simulink model "TosAeb"  (R2021a)
settings: Solver=FixedStepDiscrete  StartTime=0.0  StopTime=inf  FixedStep=0.01  RelTol=1e-3
undefined symbols (not defined in the file or in its folder): ACCModSts, AccSts1, AEB_ObjectMovingOrientation, AEB_ObjectMovingState, CN_BTCActive, CN_CurrentGearR, CN_CurrentGearR_DHT, CN_PTCActive, CN_VDCActive, CN_VSC_EspOff, CN_VSC_FCW_AEB_Error, CN_VSC_FCW_AEB_NoDispay, CN_VSC_FCW_AEB_PerfmDgdtn, CN_VSC_FCW_AEB_TempUnAvlbl, CN_VSC_VehStandstill, CNaccelAvoidMax, CNaccelAvoidMin, CNbrakePedalPress, CNcomfortStrLeftAccelMax, CNcomfortStrLeftAccelMin, CNcomfortStrRightAccelMax, CNcomfortStrRightAccelMin, CNDrvDoorOpen, CNDrvModeEco, CNDrvModeNorm, CNDrvModeOther, CNDrvModeSport, CNDrvSBRreminder, CNNull, CNobjInvalidID, CNPassDoorOpen, CNPassSBRreminder, CNPEBSAccelNegInf, CNPEBSAccelNull, CNPEBSTimeInf, CNPEBSTimeNull, CNSTOPMAX, CNSTOPNULL, CNsubjWidth, CNSwtOFF, CNSwtON, CNTTCMAX, CNTTCNULL, CNVehConfigAEBCycNone, CNVehConfigAEBNone, CNVehConfigAEBPedNone, CNVehConfigFCWNone, CNVehConfigJANone, CollisionSeverity, FALSE, FCWSnvty, JACycObjCalcInfoDefaultParams, JAPedObjCalcInfoDefaultParams, JAVehObjCalcInfoDefaultParams, latCycObjCalcInfoDefaultParams, latPedObjCalcInfoDefaultParams, longObjCalcInfoDefaultParams, ModSts, movingStatus, ObjectType ... (+1112)
libraries: simulink x3031, AEBSFunctionLib x50, Ta_Library x33, AEBSStateMachineLib x23, Mylibrary x12, simulink_extras x7, ObjSelect_lib x3, dspstat3 x2
(part 22 of 102)

== system TosAeb/AEB_Objsel/TA_Processor/PreProcessor/VehiclePreProcessor/PathEstimn_Calculate/For Iterator PahEstimn/SetVehicleStateAtT0 ==
  VehSelf : Inport
  Curvature : Inport  Port=2
  CurvatureRate : Inport  Port=3
  Index : Inport  Port=4
  VehicleMotionT1 : Inport  Port=5
  Bus Creator1 : BusCreator  Inputs=11
  Bus Selector : BusSelector  OutputSignals=Veh_LgtSpd_mps,Veh_LgtAccel_mps2
  Bus Selector1 : BusSelector  OutputSignals=PositionLongitudinal
  Bus Selector10 : BusSelector  OutputSignals=HeadingAngle
  Bus Selector2 : BusSelector  OutputSignals=VelocityLateral
  Bus Selector3 : BusSelector  OutputSignals=A
  Bus Selector4 : BusSelector  OutputSignals=AccelerationLongitudinal
  Bus Selector5 : BusSelector  OutputSignals=PositionLateral
  Bus Selector6 : BusSelector  OutputSignals=VelocityLateral
  Bus Selector7 : BusSelector  OutputSignals=AccelerationLateral
  Bus Selector8 : BusSelector  OutputSignals=Speed
  Bus Selector9 : BusSelector  OutputSignals=Curvature
  Compare To Constant : simulink/Logic and Bit Operations/Compare To Constant  relop===  const=1  OutDataTypeStr=boolean
  Constant{,1..4} (5 alike) : Constant  Value=single(0)
  Multiport Switch : MultiPortSwitch  Inputs=4
  Product : Product  Inputs=3
  Switch{,1..9} (10 alike) : Switch  Criteria=u2 ~= 0
  Unary Minus : UnaryMinus
  Unit Delay{,1..9} (10 alike) : UnitDelay  SampleTime=-1  HasFrameUpgradeWarning=on
  VehicleMotionT0 : Outport
  wires:
  Index/1 -> [Index], Compare To Constant/1
  Compare To Constant/1 -> [InitialLoop]
  Constant/1 -> Switch/1
  [InitialLoop] -> Switch/2
  VehicleMotionT1/1 -> [VehicleMotionT1]
  Bus Selector1/1 -> Unit Delay/1  "<PositionLongitudinal>"
  Unit Delay/1 -> Switch/3
  [InitialLoop] -> Switch1/2
  [VLgt] -> Switch1/1
  Unit Delay1/1 -> Switch1/3
  [InitialLoop] -> Switch2/2
  [ALgt] -> Switch2/1
  Unit Delay2/1 -> Switch2/3
  [VehicleMotionT1] -> Bus Selector2/1  "VelocityLongItudal"
  [VehicleMotionT1] -> Bus Selector3/1  "A"
  [InitialLoop] -> Switch3/2
  [ALgt] -> Switch3/1
  [VehicleMotionT1] -> Bus Selector4/1  "ALgt"
  Bus Selector4/1 -> Unit Delay3/1  "<AccelerationLongitudinal>"
  Unit Delay3/1 -> Switch3/3
  [VehicleMotionT1] -> Bus Selector5/1  "PosnLat"
  Bus Selector5/1 -> Unit Delay4/1  "<PositionLateral>"
  Unit Delay4/1 -> Switch4/3
  [InitialLoop] -> Switch4/2
  Constant1/1 -> Switch4/1
  [VehicleMotionT1] -> Bus Selector6/1  "VLat"
  Unit Delay5/1 -> Switch5/3
  [InitialLoop] -> Switch5/2
  Constant2/1 -> Switch5/1
  Product/1 -> Switch6/1
  [InitialLoop] -> Switch6/2
  Unit Delay6/1 -> Switch6/3
  [VehicleMotionT1] -> Bus Selector7/1  "ALat"
  [VLgt] -> Product/2, Product/3
  Curvature/1 -> Switch9/1, Product/1
  [Index] -> Multiport Switch/1
  CurvatureRate/1 -> Multiport Switch/2, Unary Minus/1
  Unary Minus/1 -> Multiport Switch/3
  Constant3/1 -> Multiport Switch/4, Multiport Switch/5
  Constant4/1 -> Switch7/1
  [InitialLoop] -> Switch7/2
  Bus Selector7/1 -> Unit Delay6/1  "<AccelerationLateral>"
  [VehicleMotionT1] -> Bus Selector10/1  "HeadingAngle"
  Bus Selector10/1 -> Unit Delay9/1  "<HeadingAngle>"
  [VLgt] -> Switch8/1
  [InitialLoop] -> Switch8/2
  [VehicleMotionT1] -> Bus Selector8/1  "Speed"
  Bus Selector8/1 -> Unit Delay7/1  "<Speed>"
  [InitialLoop] -> Switch9/2
  [VehicleMotionT1] -> Bus Selector9/1  "Curvature"
  Bus Selector9/1 -> Unit Delay8/1  "<Curvature>"
  Switch7/1 -> Bus Creator1/11  "HeadingAngle"
  Switch2/1 -> Bus Creator1/3  "A"
  Switch3/1 -> Bus Creator1/4  "AccelerationLongitudinal"
  Switch4/1 -> Bus Creator1/5  "PositionLateral"
  Switch6/1 -> Bus Creator1/7  "AccelerationLateral"
  Switch8/1 -> Bus Creator1/8  "Speed"
  Switch9/1 -> Bus Creator1/9  "Curvature"
  Multiport Switch/1 -> Bus Creator1/10  "CurvatureRate"
  Switch/1 -> Bus Creator1/1  "PositionLongitudinal"
  Switch1/1 -> Bus Creator1/2  "VelocityLongitudinal"
  Switch5/1 -> Bus Creator1/6  "VelocityLateral"
  Bus Creator1/1 -> VehicleMotionT0/1  "VehicleMotionT0"
  VehSelf/1 -> Bus Selector/1  "1"
  [VehicleMotionT1] -> Bus Selector1/1  "PositionLongItudal"
  Bus Selector2/1 -> Unit Delay1/1  "<VelocityLateral>"
  Bus Selector3/1 -> Unit Delay2/1  "<A>"
  Bus Selector6/1 -> Unit Delay5/1  "<VelocityLateral>"
  Bus Selector/1 -> [VLgt]  "<Veh_LgtSpd_mps>"
  Bus Selector/2 -> [ALgt]  "<Veh_LgtAccel_mps2>"
  Unit Delay7/1 -> Switch8/3
  Unit Delay8/1 -> Switch9/3
  Unit Delay9/1 -> Switch7/3

== system TosAeb/ELK_Objsel/Target_Selection/If Action Subsystem/ELK_TargetSelection/If Action Threat_Assessment_Left/First_Order_Lag_Filter/Subsystem ==
  note: Filter Gain = T / (T + t)
      | T=> Loop time and
      | t => time constant
  CL_TIME : Inport
  time_constant : Inport  Port=2
  Product : Product  Description=_GWMAB30P4.LKAFN.000_144_  Inputs=*/  InputSameDT=on  OutDataTypeStr=Inherit: Same as first input
  Sum2 : Sum  Description=_GWMAB30P4.LKAFN.000_145_  InputSameDT=on  OutDataTypeStr=Inherit: Same as first input
  Gain : Outport
  wires:
  time_constant/1 -> Sum2/2
  CL_TIME/1 -> Sum2/1, Product/1
  Product/1 -> Gain/1
  Sum2/1 -> Product/2

== system TosAeb/ELK_Objsel/Target_Selection/If Action Subsystem/ELK_TargetSelection/If Action Threat_Assessment_Left/First_Order_Lag_Filter1/Subsystem ==
  note: Filter Gain = T / (T + t)
      | T=> Loop time and
      | t => time constant
  CL_TIME : Inport
  time_constant : Inport  Port=2
  Product : Product  Description=_GWMAB30P4.LKAFN.000_144_  Inputs=*/  InputSameDT=on  OutDataTypeStr=Inherit: Same as first input
  Sum2 : Sum  Description=_GWMAB30P4.LKAFN.000_145_  InputSameDT=on  OutDataTypeStr=Inherit: Same as first input
  Gain : Outport
  wires:
  Sum2/1 -> Product/2
  Product/1 -> Gain/1
  CL_TIME/1 -> Product/1, Sum2/1
  time_constant/1 -> Sum2/2

== system TosAeb/ELK_Objsel/Target_Selection/If Action Subsystem/ELK_TargetSelection/If Action Threat_Assessment_Left1/First_Order_Lag_Filter/Subsystem ==
  note: Filter Gain = T / (T + t)
      | T=> Loop time and
      | t => time constant
  CL_TIME : Inport
  time_constant : Inport  Port=2
  Product : Product  Description=_GWMAB30P4.LKAFN.000_144_  Inputs=*/  InputSameDT=on  OutDataTypeStr=Inherit: Same as first input
  Sum2 : Sum  Description=_GWMAB30P4.LKAFN.000_145_  InputSameDT=on  OutDataTypeStr=Inherit: Same as first input
  Gain : Outport
  wires:
  Sum2/1 -> Product/2
  Product/1 -> Gain/1
  CL_TIME/1 -> Product/1, Sum2/1
  time_constant/1 -> Sum2/2

== system TosAeb/ELK_Objsel/Target_Selection/If Action Subsystem/ELK_TargetSelection/If Action Threat_Assessment_Left1/First_Order_Lag_Filter1/Subsystem ==
  note: Filter Gain = T / (T + t)
      | T=> Loop time and
      | t => time constant
  CL_TIME : Inport
  time_constant : Inport  Port=2
  Product : Product  Description=_GWMAB30P4.LKAFN.000_144_  Inputs=*/  InputSameDT=on  OutDataTypeStr=Inherit: Same as first input
  Sum2 : Sum  Description=_GWMAB30P4.LKAFN.000_145_  InputSameDT=on  OutDataTypeStr=Inherit: Same as first input
  Gain : Outport
  wires:
  time_constant/1 -> Sum2/2
  CL_TIME/1 -> Sum2/1, Product/1
  Product/1 -> Gain/1
  Sum2/1 -> Product/2

== system TosAeb/ELK_Objsel/Target_Selection/If Action Subsystem/ELK_TargetSelection/If Action Threat_Assessment_Right/First_Order_Lag_Filter/Subsystem ==
  note: Filter Gain = T / (T + t)
      | T=> Loop time and
      | t => time constant
  CL_TIME : Inport
  time_constant : Inport  Port=2
  Product : Product  Description=_GWMAB30P4.LKAFN.000_144_  Inputs=*/  InputSameDT=on  OutDataTypeStr=Inherit: Same as first input
  Sum2 : Sum  Description=_GWMAB30P4.LKAFN.000_145_  InputSameDT=on  OutDataTypeStr=Inherit: Same as first input
  Gain : Outport
  wires:
  time_constant/1 -> Sum2/2
  CL_TIME/1 -> Sum2/1, Product/1
  Product/1 -> Gain/1
  Sum2/1 -> Product/2

== system TosAeb/ELK_Objsel/Target_Selection/If Action Subsystem/ELK_TargetSelection/If Action Threat_Assessment_Right/First_Order_Lag_Filter1/Subsystem ==
  note: Filter Gain = T / (T + t)
      | T=> Loop time and
      | t => time constant
  CL_TIME : Inport
  time_constant : Inport  Port=2
  Product : Product  Description=_GWMAB30P4.LKAFN.000_144_  Inputs=*/  InputSameDT=on  OutDataTypeStr=Inherit: Same as first input
  Sum2 : Sum  Description=_GWMAB30P4.LKAFN.000_145_  InputSameDT=on  OutDataTypeStr=Inherit: Same as first input
  Gain : Outport
  wires:
  Sum2/1 -> Product/2
  Product/1 -> Gain/1
  CL_TIME/1 -> Product/1, Sum2/1
  time_constant/1 -> Sum2/2

== system TosAeb/ELK_Objsel/Target_Selection/If Action Subsystem/ELK_TargetSelection/If Action Threat_Assessment_Right1/First_Order_Lag_Filter/Subsystem ==
  note: Filter Gain = T / (T + t)
      | T=> Loop time and
      | t => time constant
  CL_TIME : Inport
  time_constant : Inport  Port=2
  Product : Product  Description=_GWMAB30P4.LKAFN.000_144_  Inputs=*/  InputSameDT=on  OutDataTypeStr=Inherit: Same as first input
  Sum2 : Sum  Description=_GWMAB30P4.LKAFN.000_145_  InputSameDT=on  OutDataTypeStr=Inherit: Same as first input
  Gain : Outport
  wires:
  Sum2/1 -> Product/2
  Product/1 -> Gain/1
  CL_TIME/1 -> Product/1, Sum2/1
  time_constant/1 -> Sum2/2

== system TosAeb/ELK_Objsel/Target_Selection/If Action Subsystem/ELK_TargetSelection/If Action Threat_Assessment_Right1/First_Order_Lag_Filter1/Subsystem ==
  note: Filter Gain = T / (T + t)
      | T=> Loop time and
      | t => time constant
  CL_TIME : Inport
  time_constant : Inport  Port=2
  Product : Product  Description=_GWMAB30P4.LKAFN.000_144_  Inputs=*/  InputSameDT=on  OutDataTypeStr=Inherit: Same as first input
  Sum2 : Sum  Description=_GWMAB30P4.LKAFN.000_145_  InputSameDT=on  OutDataTypeStr=Inherit: Same as first input
  Gain : Outport
  wires:
  time_constant/1 -> Sum2/2
  CL_TIME/1 -> Sum2/1, Product/1
  Product/1 -> Gain/1
  Sum2/1 -> Product/2

== system TosAeb/FcwAebJa_Step_sys/ALG_AEBSystem/ALG_AEBController/AEB_FuncCondCalcApp/CycFunctionCondCalc/cycAEBfullSuppAborCond/cycAEBfullAborCondNoStandstillCalc ==
  inputSignals : Inport
  Bus Selector : BusSelector  OutputSignals=latCycTgtCalcInfo.latCycObjCalcInfo.latCycObjRawInfo.ObjectID,latCycTgtCalcInfo.latCycObjCalcInfo.latCycObjRawInfo.ObjectTypeCameraConfirmed,latCycTgtCalcInfo.latCycObjCalcInfo.CollisionRiskProb
  Bus Selector1 : BusSelector  OutputSignals=vehCanInfo.SteerWheelAngle,vehCanInfo.SteerWheelSpd,vehStateCalcInfo.VSCPedFuncSuppAborCond.VSCPedAEBfullAborCond,latFuncActiveStateTime.pedAEBfullWorkTime,vehStateCalcInfo.VSCVehStandStill
  Bus Selector2 : BusSelector  OutputSignals=latCycTgtCalcInfo.latCycCollisionAssessInfo.latCollisionSeverity,latCycTgtCalcInfo.latCycObjCalcInfo.CollisionRiskProb,subjInfo.subjVel,latCycTgtCalcInfo.latCycObjCalcInfo.latCycObjRawInfo.dy,latCycTgtCalcInfo.latCycObjCalcInfo.latCycObjRawInfo.dx,latCycTgtCalcInfo.latCycObjCalcInfo.latCycObjRawInfo.ObjectMovingState
  Ground : Ground
  cycAEBfullAborCondFinalJudge : Subsystem
  cycAEBfullObjRelActiveCondCalc : Subsystem
  cycAEBfullSituationRelAborCondCalc : Subsystem
  cycAEBfullVehRelAborCondCalc : Subsystem
  cycAEBfullAborCondNoStandstillFulfilled : Outport
  wires:
  Bus Selector2/2 -> cycAEBfullSituationRelAborCondCalc/3(objLatCollsionRiskProb)  "<CollisionRiskProb>"
  Bus Selector2/3 -> cycAEBfullSituationRelAborCondCalc/4(subjVel)  "<subjVel>"
  Bus Selector2/4 -> cycAEBfullSituationRelAborCondCalc/5(dy)  "<dy>"
  Bus Selector2/5 -> cycAEBfullSituationRelAborCondCalc/6(dx)  "<dx>"
  Bus Selector2/6 -> cycAEBfullSituationRelAborCondCalc/7(ObjectMovingState)  "<ObjectMovingState>"
  cycAEBfullObjRelActiveCondCalc/1(cycAEBfullObjRelAborCondFulfilled) -> cycAEBfullAborCondFinalJudge/1(cycAEBfullObjRelAborCondFulfilled)  "AEB_cycAEBfullObjRelAborCond"
  cycAEBfullVehRelAborCondCalc/1(cycAEBfullVehRelAborCondFulfilled) -> cycAEBfullAborCondFinalJudge/2(cycAEBfullVehRelAborCondFulfilled)  "AEB_cycAEBfullVehRelAborCond"
  cycAEBfullSituationRelAborCondCalc/1(cycAEBfullSituationRelAborCondFulfilled) -> cycAEBfullAborCondFinalJudge/3(cycAEBfullSituationRelAborCondFulfilled)  "AEB_cycAEBfullSitRelAborCond"
  cycAEBfullAborCondFinalJudge/1(cycAEBfullAborCondNoStandstillFulfilled) -> cycAEBfullAborCondNoStandstillFulfilled/1
  inputSignals/1 -> Bus Selector/1, Bus Selector2/1, Bus Selector1/1
  Bus Selector1/1 -> cycAEBfullVehRelAborCondCalc/1(SteerWheelAngle)  "<SteerWheelAngle>"
  Bus Selector1/2 -> cycAEBfullVehRelAborCondCalc/2(SteerWheelSpd)  "<SteerWheelSpd>"
  Bus Selector1/3 -> cycAEBfullVehRelAborCondCalc/3(vehStateCalcAEBfullAborFlg)  "<VSCPedAEBfullAborCond>"
  Bus Selector1/4 -> cycAEBfullVehRelAborCondCalc/4(cycAEBfullWorkTime)  "<pedAEBfullWorkTime>"
  Bus Selector1/5 -> cycAEBfullVehRelAborCondCalc/5(vehStateCalcStandstill)  "<VSCVehStandStill>"
  Ground/1 -> cycAEBfullSituationRelAborCondCalc/1(cycVehVelReduced)
  Bus Selector2/1 -> cycAEBfullSituationRelAborCondCalc/2(cycCollisionSeverity)  "<latCollisionSeverity>"
  Bus Selector/1 -> cycAEBfullObjRelActiveCondCalc/1(ObjectID)  "<ObjectID>"
  Bus Selector/2 -> cycAEBfullObjRelActiveCondCalc/2(objIsFusionTarget)  "<ObjectTypeCameraConfirmed>"
  Bus Selector/3 -> cycAEBfullObjRelActiveCondCalc/3(latCollsionRiskProb)  "<CollisionRiskProb>"

== system TosAeb/FcwAebJa_Step_sys/ALG_AEBSystem/ALG_AEBController/AEB_FuncCondCalcApp/CycFunctionCondCalc/cycAEBfullSuppAborCond/cycAEBfullAborCondStandstillCalc ==
  inputSignals : Inport
  Bus Selector : BusSelector  OutputSignals=subjInfo.subjVel,vehCanInfo.SteerWheelAngle,vehCanInfo.SteerWheelSpd,vehStateCalcInfo.VSCCycFuncSuppAborCond.VSCcycAEBfullAborCond,latFuncActiveStateTime.cycAEBfullWorkTime,vehStateCalcInfo.VSCVehStandStill
  cycAEBfullVehRelAborCondCalc : Subsystem
  cycAEBfullAborCondStandstillFulfilled : Outport
  wires:
  cycAEBfullVehRelAborCondCalc/1(cycAEBfullVehRelAborCondFulfilled) -> cycAEBfullAborCondStandstillFulfilled/1
  inputSignals/1 -> Bus Selector/1
  Bus Selector/3 -> cycAEBfullVehRelAborCondCalc/3(SteerWheelSpd)  "<SteerWheelSpd>"
  Bus Selector/2 -> cycAEBfullVehRelAborCondCalc/2(SteerWheelAngle)  "<SteerWheelAngle>"
  Bus Selector/4 -> cycAEBfullVehRelAborCondCalc/4(vehStateCalcAEBfullAborFlg)  "<VSCcycAEBfullAborCond>"
  Bus Selector/1 -> cycAEBfullVehRelAborCondCalc/1(subjVel)  "<subjVel>"
  Bus Selector/5 -> cycAEBfullVehRelAborCondCalc/5(cycAEBfullWorkTime)  "<cycAEBfullWorkTime>"
  Bus Selector/6 -> cycAEBfullVehRelAborCondCalc/6(vehStateCalcStandstill)  "<VSCVehStandStill>"

== system TosAeb/FcwAebJa_Step_sys/ALG_AEBSystem/ALG_AEBController/AEB_FuncCondCalcApp/CycFunctionCondCalc/cycAEBfullSuppAborCond/cycAEBfullSuppCondCalc ==
  inputSignals : Inport
  Bus Selector : BusSelector  OutputSignals=latCycTgtCalcInfo.latCycObjCalcInfo.latCycObjRawInfo.ObjectID,latCycTgtCalcInfo.latCycObjCalcInfo.latCycObjAbsInfo.vxAbsObj,latCycTgtCalcInfo.latCycObjCalcInfo.latCycObjAbsInfo.ObjFusQuality,latCycTgtCalcInfo.latCycObjCalcInfo.latCycObjRawInfo.dy,latCycTgtCalcInfo.latCycObjCalcInfo.latCycObjRawInfo.vy
  Bus Selector1 : BusSelector  OutputSignals=subjInfo.subjVel,vehConfigInfo.VehConfigIbooster,vehCanInfo.ESP_MasterCylindBrakePressValid,vehCanInfo.ESP_MasterCylindBrakePress,vehCanInfo.SteerWheelAngle,vehCanInfo.SteerWheelSpd,vehCanInfo.AccrPedlPosnDiagc,vehStateCalcInfo.VSCCycFuncSuppAborCond.VSCcycAEBfullSuppCond
  Bus Selector2 : BusSelector  OutputSignals=latCycTgtCalcInfo.latCycCollisionAssessInfo.latCollisionSeverity,subjInfo.subjCurvTraj,subjInfo.subjLatAccel,latCycTgtCalcInfo.latCycObjCalcInfo.CollisionRiskProb,latCycTgtCalcInfo.latCycObjCalcInfo.PreCollisionFlg,latCycTgtCalcInfo.latCycObjCalcInfo.latCycObjRawInfo.ObjectTypeCameraConfirmed,latCycTgtCalcInfo.latCycObjCalcInfo.latCycObjRawInfo.vy,latCycTgtCalcInfo.latCycObjCalcInfo.latCycObjAbsInfo.dxFrontBumper,latCycTgtCalcInfo.latCycObjCalcInfo.latCycObjRawInfo.dy,vehStateCalcInfo.VSC_AEBFuncSwtSts.FCWSnvty,subjInfo.subjVel,latCycTgtCalcInfo.latCycCollisionAssessInfo.distBraking4FullBrake,latCycTgtCalcInfo.latCycCollisionAssessInfo.distSecure
  cycAEBfullObjRelSuppCondCalc : Subsystem
  cycAEBfullSituationRelSuppCondCalc : Subsystem
  cycAEBfullSuppCondFinalJudge : Subsystem
  cycAEBfullVehRelSuppCondCalc : Subsystem
  cycAEBfullSuppCondFulfilled : Outport
  wires:
  Bus Selector1/2 -> cycAEBfullVehRelSuppCondCalc/2(VehConfigIbooster)  "<VehConfigIbooster>"
  Bus Selector/5 -> cycAEBfullObjRelSuppCondCalc/5(vy)  "<vy>"
  Bus Selector/4 -> cycAEBfullObjRelSuppCondCalc/4(dy)  "<dy>"
  Bus Selector/3 -> cycAEBfullObjRelSuppCondCalc/3(ObjFusQuality)  "<ObjFusQuality>"
  Bus Selector/2 -> cycAEBfullObjRelSuppCondCalc/2(vxAbsObj)  "<vxAbsObj>"
  Bus Selector2/13 -> cycAEBfullSituationRelSuppCondCalc/13(distSecure)  "<distSecure>"
  Bus Selector2/12 -> cycAEBfullSituationRelSuppCondCalc/12(distBraking4FullBrake)  "<distBraking4FullBrake>"
  Bus Selector2/11 -> cycAEBfullSituationRelSuppCondCalc/11(subVel)  "<subjVel>"
  Bus Selector2/10 -> cycAEBfullSituationRelSuppCondCalc/10(FcwHMISetting)  "<FCWSnvty>"
  Bus Selector2/9 -> cycAEBfullSituationRelSuppCondCalc/9(dy)  "<dy>"
  Bus Selector2/8 -> cycAEBfullSituationRelSuppCondCalc/8(dxFrontBumper)  "<dxFrontBumper>"
  Bus Selector2/7 -> cycAEBfullSituationRelSuppCondCalc/7(vy)  "<vy>"
  Bus Selector2/6 -> cycAEBfullSituationRelSuppCondCalc/6(ObjectTypeCameraConfirmed)  "<ObjectTypeCameraConfirmed>"
  Bus Selector2/5 -> cycAEBfullSituationRelSuppCondCalc/5(cycCollisionProbFlg)  "<PreCollisionFlg>"
  Bus Selector2/4 -> cycAEBfullSituationRelSuppCondCalc/4(cycCollisionRisk)  "<CollisionRiskProb>"
  Bus Selector2/3 -> cycAEBfullSituationRelSuppCondCalc/3(subjLatAccel)  "<subjLatAccel>"
  Bus Selector2/1 -> cycAEBfullSituationRelSuppCondCalc/1(latCollisionSeverity)  "<latCollisionSeverity>"
  Bus Selector2/2 -> cycAEBfullSituationRelSuppCondCalc/2(subjCurvTraj)  "<subjCurvTraj>"
  cycAEBfullSuppCondFinalJudge/1(cycAEBfullSuppCondFulfilled) -> cycAEBfullSuppCondFulfilled/1
  cycAEBfullVehRelSuppCondCalc/1(cycAEBfullVehRelSuppCondFulfilled) -> cycAEBfullSuppCondFinalJudge/2(cycAEBfullVehRelSuppCondFulfilled)  "AEB_cycAEBfullVehRelSuppCond"
  cycAEBfullObjRelSuppCondCalc/1(cycAEBfullObjRelSuppCondFulfilled) -> cycAEBfullSuppCondFinalJudge/1(cycAEBfullObjRelSuppCondFulfilled)  "AEB_cycAEBfullObjRelSuppCond"
  cycAEBfullSituationRelSuppCondCalc/1(cycAEBfullSituationRelSuppCondFulfilled) -> cycAEBfullSuppCondFinalJudge/3(cycAEBfullSituationRelSuppCondFulfilled)  "AEB_cycAEBfullSitRelSuppCond"
  inputSignals/1 -> Bus Selector/1, Bus Selector1/1, Bus Selector2/1
  Bus Selector/1 -> cycAEBfullObjRelSuppCondCalc/1(ObjectID)  "<ObjectID>"
  Bus Selector1/1 -> cycAEBfullVehRelSuppCondCalc/1(subjVel)  "<subjVel>"
  Bus Selector1/3 -> cycAEBfullVehRelSuppCondCalc/3(ESP_MasterCylindBrakePressValid)  "<ESP_MasterCylindBrakePressValid>"
  Bus Selector1/4 -> cycAEBfullVehRelSuppCondCalc/4(ESP_MasterCylindBrakePress)  "<ESP_MasterCylindBrakePress>"
  Bus Selector1/5 -> cycAEBfullVehRelSuppCondCalc/5(SteerWheelAngle)  "<SteerWheelAngle>"
  Bus Selector1/6 -> cycAEBfullVehRelSuppCondCalc/6(SteerWheelSpd)  "<SteerWheelSpd>"
  Bus Selector1/7 -> cycAEBfullVehRelSuppCondCalc/7(AccrCyclPosnDiagc)  "<AccrPedlPosnDiagc>"
  Bus Selector1/8 -> cycAEBfullVehRelSuppCondCalc/8(vehStateCalcCycAEBfullSuppFlg)  "<VSCcycAEBfullSuppCond>"

== system TosAeb/FcwAebJa_Step_sys/ALG_AEBSystem/ALG_AEBController/AEB_FuncCondCalcApp/CycFunctionCondCalc/cycAEBpartSuppAborCond/cycAEBpartAborCondNoStandstillCalc ==
  inputSignals : Inport
  Bus Selector : BusSelector  OutputSignals=latCycTgtCalcInfo.latCycObjCalcInfo.latCycObjRawInfo.ObjectID,latCycTgtCalcInfo.latCycObjCalcInfo.latCycObjRawInfo.ObjectTypeCameraConfirmed,latCycTgtCalcInfo.latCycObjCalcInfo.CollisionRiskProb
  Bus Selector1 : BusSelector  OutputSignals=vehCanInfo.SteerWheelAngle,vehCanInfo.SteerWheelSpd,vehStateCalcInfo.VSCCycFuncSuppAborCond.VSCcycAEBpartAborCond
  Bus Selector2 : BusSelector  OutputSignals=latCycTgtCalcInfo.latCycCollisionAssessInfo.latCollisionSeverity,latCycTgtCalcInfo.latCycObjCalcInfo.CollisionRiskProb,subjInfo.subjVel,latCycTgtCalcInfo.latCycObjCalcInfo.latCycObjRawInfo.dy,latCycTgtCalcInfo.latCycObjCalcInfo.latCycObjRawInfo.dx,latCycTgtCalcInfo.latCycObjCalcInfo.latCycObjRawInfo.ObjectMovingState
  Ground : Ground
  cycAEBpartAborCondFinalJudge : Subsystem
  cycAEBpartObjRelActiveCondCalc : Subsystem
  cycAEBpartSituationRelAborCondCalc : Subsystem
  cycAEBpartVehRelAborCondCalc : Subsystem
  cycAEBpartAborCondNoStandstillFulfilled : Outport
  wires:
  Bus Selector2/6 -> cycAEBpartSituationRelAborCondCalc/7(ObjectMovingState)  "<ObjectMovingState>"
  Bus Selector2/5 -> cycAEBpartSituationRelAborCondCalc/6(dx)  "<dx>"
  Bus Selector2/1 -> cycAEBpartSituationRelAborCondCalc/2(latCollisionSeverity)  "<latCollisionSeverity>"
  Bus Selector2/2 -> cycAEBpartSituationRelAborCondCalc/3(CollsionRiskProb)  "<CollisionRiskProb>"
  Bus Selector2/3 -> cycAEBpartSituationRelAborCondCalc/4(subjVel)  "<subjVel>"
  Bus Selector2/4 -> cycAEBpartSituationRelAborCondCalc/5(dy)  "<dy>"
  Bus Selector1/3 -> cycAEBpartVehRelAborCondCalc/3(vehStateCalcAEBpartAborFlg)  "<VSCcycAEBpartAborCond>"
  Bus Selector1/2 -> cycAEBpartVehRelAborCondCalc/2(SteerWheelSpd)  "<SteerWheelSpd>"
  Bus Selector1/1 -> cycAEBpartVehRelAborCondCalc/1(SteerWheelAngle)  "<SteerWheelAngle>"
  cycAEBpartObjRelActiveCondCalc/1(cycAEBpartObjRelAborCondFulfilled) -> cycAEBpartAborCondFinalJudge/1(cycAEBpartObjRelAborCondFulfilled)  "AEB_cycAEBpartObjRelAborCond"
  cycAEBpartVehRelAborCondCalc/1(cycAEBpartVehRelAborCondFulfilled) -> cycAEBpartAborCondFinalJudge/2(cycAEBpartVehRelAborCondFulfilled)  "AEB_cycAEBpartVehRelAborCond"
  cycAEBpartSituationRelAborCondCalc/1(cycAEBpartSituationRelAborCondFulfilled) -> cycAEBpartAborCondFinalJudge/3(cycAEBpartSituationRelAborCondFulfilled)  "AEB_cycAEBpartSitRelAborCond"
  cycAEBpartAborCondFinalJudge/1(cycAEBpartAborCondNoStandstillFulfilled) -> cycAEBpartAborCondNoStandstillFulfilled/1
  inputSignals/1 -> Bus Selector/1, Bus Selector2/1, Bus Selector1/1
  Ground/1 -> cycAEBpartSituationRelAborCondCalc/1(cycVehVelReduced)
  Bus Selector/1 -> cycAEBpartObjRelActiveCondCalc/1(ObjectID)  "<ObjectID>"
  Bus Selector/2 -> cycAEBpartObjRelActiveCondCalc/2(objIsFusionTarget)  "<ObjectTypeCameraConfirmed>"
  Bus Selector/3 -> cycAEBpartObjRelActiveCondCalc/3(latCollsionRiskProb)  "<CollisionRiskProb>"

== system TosAeb/FcwAebJa_Step_sys/ALG_AEBSystem/ALG_AEBController/AEB_FuncCondCalcApp/CycFunctionCondCalc/cycAEBpartSuppAborCond/cycAEBpartAborCondStandstillCalc ==
  inputSignals : Inport
  Bus Selector : BusSelector  OutputSignals=vehCanInfo.SteerWheelAngle,vehCanInfo.SteerWheelSpd,vehStateCalcInfo.VSCCycFuncSuppAborCond.VSCcycAEBpartAborCond
  cycAEBpartVehRelAborCondCalc : Subsystem
  cycAEBpartAborCondStandstillFulfilled : Outport
  wires:
  Bus Selector/3 -> cycAEBpartVehRelAborCondCalc/3(vehStateCalcAEBpartAborFlg)  "<VSCcycAEBpartAborCond>"
  Bus Selector/1 -> cycAEBpartVehRelAborCondCalc/1(SteerWheelAngle)  "<SteerWheelAngle>"
  Bus Selector/2 -> cycAEBpartVehRelAborCondCalc/2(SteerWheelSpd)  "<SteerWheelSpd>"
  cycAEBpartVehRelAborCondCalc/1(cycAEBpartVehRelAborCondFulfilled) -> cycAEBpartAborCondStandstillFulfilled/1
  inputSignals/1 -> Bus Selector/1

== system TosAeb/FcwAebJa_Step_sys/ALG_AEBSystem/ALG_AEBController/AEB_FuncCondCalcApp/CycFunctionCondCalc/cycAEBpartSuppAborCond/cycAEBpartSuppCondCalc ==
  inputSignals : Inport
  Bus Selector : BusSelector  OutputSignals=latCycTgtCalcInfo.latCycObjCalcInfo.latCycObjRawInfo.ObjectID,latCycTgtCalcInfo.latCycObjCalcInfo.latCycObjAbsInfo.vxAbsObj,latCycTgtCalcInfo.latCycObjCalcInfo.latCycObjAbsInfo.ObjFusQuality,latCycTgtCalcInfo.latCycObjCalcInfo.latCycObjRawInfo.dy,latCycTgtCalcInfo.latCycObjCalcInfo.latCycObjRawInfo.vy
  Bus Selector1 : BusSelector  OutputSignals=subjInfo.subjVel,vehConfigInfo.VehConfigIbooster,vehCanInfo.ESP_MasterCylindBrakePressValid,vehCanInfo.ESP_MasterCylindBrakePress,vehCanInfo.IB_BrkPedalAppld,vehCanInfo.SteerWheelAngle,vehCanInfo.SteerWheelSpd,vehCanInfo.AccrPedlPosnDiagc,vehStateCalcInfo.VSCCycFuncSuppAborCond.VSCcycAEBpartSuppCond
  Bus Selector2 : BusSelector  OutputSignals=latCycTgtCalcInfo.latCycCollisionAssessInfo.latCollisionSeverity,subjInfo.subjCurvTraj,subjInfo.subjLatAccel,latCycTgtCalcInfo.latCycObjCalcInfo.latCycObjRawInfo.ObjectTypeCameraConfirmed,latCycTgtCalcInfo.latCycObjCalcInfo.CollisionRiskProb,latCycTgtCalcInfo.latCycObjCalcInfo.PreCollisionFlg,latCycTgtCalcInfo.latCycObjCalcInfo.latCycObjRawInfo.vy,latCycTgtCalcInfo.latCycObjCalcInfo.latCycObjAbsInfo.dxFrontBumper,latCycTgtCalcInfo.latCycCollisionAssessInfo.distBraking4PartBrake,latCycTgtCalcInfo.latCycCollisionAssessInfo.distSecure,subjInfo.subjVel,latCycTgtCalcInfo.latCycObjCalcInfo.latCycObjRawInfo.dy,vehStateCalcInfo.VSC_AEBFuncSwtSts.FCWSnvty
  cycAEBpartObjRelActiveCondCalc : Subsystem
  cycAEBpartSituationRelActiveCondCalc : Subsystem
  cycAEBpartSuppCondFinalJudge : Subsystem
  cycAEBpartVehRelActiveCondCalc : Subsystem
  cycAEBpartSuppCondFulfilled : Outport
  wires:
  Bus Selector1/2 -> cycAEBpartVehRelActiveCondCalc/2(VehConfigIbooster)  "<VehConfigIbooster>"
  Bus Selector/5 -> cycAEBpartObjRelActiveCondCalc/5(vy)  "<vy>"
  Bus Selector/4 -> cycAEBpartObjRelActiveCondCalc/4(dy)  "<dy>"
  Bus Selector/3 -> cycAEBpartObjRelActiveCondCalc/3(ObjFusQuality)  "<ObjFusQuality>"
  Bus Selector/2 -> cycAEBpartObjRelActiveCondCalc/2(vxAbsObj)  "<vxAbsObj>"
  Bus Selector/1 -> cycAEBpartObjRelActiveCondCalc/1(ObjectID)  "<ObjectID>"
  Bus Selector1/5 -> cycAEBpartVehRelActiveCondCalc/5(IB_BrkPedalAppld)  "<IB_BrkPedalAppld>"
  Bus Selector2/13 -> cycAEBpartSituationRelActiveCondCalc/13(FcwHMISetting)  "<FCWSnvty>"
  Bus Selector2/12 -> cycAEBpartSituationRelActiveCondCalc/12(dy)  "<dy>"
  Bus Selector2/11 -> cycAEBpartSituationRelActiveCondCalc/11(subVel)  "<subjVel>"
  Bus Selector2/10 -> cycAEBpartSituationRelActiveCondCalc/10(distSecure)  "<distSecure>"
  Bus Selector2/9 -> cycAEBpartSituationRelActiveCondCalc/9(distBraking4PartBrake)  "<distBraking4PartBrake>"
  Bus Selector2/8 -> cycAEBpartSituationRelActiveCondCalc/8(dxFrontBumper)  "<dxFrontBumper>"
  Bus Selector2/7 -> cycAEBpartSituationRelActiveCondCalc/7(vy)  "<vy>"
  Bus Selector2/6 -> cycAEBpartSituationRelActiveCondCalc/6(PreCollisionFlg)  "<PreCollisionFlg>"
  Bus Selector2/5 -> cycAEBpartSituationRelActiveCondCalc/5(colliisonRiskProb)  "<CollisionRiskProb>"
  Bus Selector2/4 -> cycAEBpartSituationRelActiveCondCalc/4(ObjectTypeCameraConfirmed)  "<ObjectTypeCameraConfirmed>"
  Bus Selector2/3 -> cycAEBpartSituationRelActiveCondCalc/3(subjLatAccel)  "<subjLatAccel>"
  Bus Selector2/2 -> cycAEBpartSituationRelActiveCondCalc/2(subjCurvTraj)  "<subjCurvTraj>"
  Bus Selector2/1 -> cycAEBpartSituationRelActiveCondCalc/1(latCollisionSeverity)  "<latCollisionSeverity>"
  cycAEBpartSituationRelActiveCondCalc/1(cycAEBpartSituationRelSuppCondFulfilled) -> cycAEBpartSuppCondFinalJudge/3(cycAEBpartSituationRelSuppCondFulfilled)  "AEB_cycAEBpartSitRelSuppCond"
  cycAEBpartObjRelActiveCondCalc/1(cycAEBpartObjRelSuppCondFulfilled) -> cycAEBpartSuppCondFinalJudge/1(cycAEBpartObjRelSuppCondFulfilled)  "AEB_cycAEBpartObjRelSuppCond"
  cycAEBpartVehRelActiveCondCalc/1(cycAEBpartVehRelSuppCondFulfilled) -> cycAEBpartSuppCondFinalJudge/2(cycAEBpartVehRelSuppCondFulfilled)  "AEB_cycAEBpartVehRelSuppCond"
  cycAEBpartSuppCondFinalJudge/1(cycAEBpartSuppCondFulfilled) -> cycAEBpartSuppCondFulfilled/1
  Bus Selector1/6 -> cycAEBpartVehRelActiveCondCalc/6(SteerWheelAngle)  "<SteerWheelAngle>"
  Bus Selector1/4 -> cycAEBpartVehRelActiveCondCalc/4(ESPmasterCylindBrakePress)  "<ESP_MasterCylindBrakePress>"
  Bus Selector1/7 -> cycAEBpartVehRelActiveCondCalc/7(SteerWheelSpd)  "<SteerWheelSpd>"
  Bus Selector1/3 -> cycAEBpartVehRelActiveCondCalc/3(ESPmasterCylindBrakePressValid)  "<ESP_MasterCylindBrakePressValid>"
  Bus Selector1/8 -> cycAEBpartVehRelActiveCondCalc/8(AccrCyclPosnDiagc)  "<AccrPedlPosnDiagc>"
  Bus Selector1/1 -> cycAEBpartVehRelActiveCondCalc/1(subjVel)  "<subjVel>"
  Bus Selector1/9 -> cycAEBpartVehRelActiveCondCalc/9(vehStateCalcCycAEBpartSuppFlg)  "<VSCcycAEBpartSuppCond>"
  inputSignals/1 -> Bus Selector/1, Bus Selector1/1, Bus Selector2/1

== system TosAeb/FcwAebJa_Step_sys/ALG_AEBSystem/ALG_AEBController/AEB_FuncCondCalcApp/CycFunctionCondCalc/cycPrefillSuppAborCond/cycPrefillAborCondCalc ==
  inputSignals : Inport
  Bus Selector : BusSelector  OutputSignals=latCycTgtCalcInfo.latCycObjCalcInfo.latCycObjRawInfo.ObjectID
  Bus Selector1 : BusSelector  OutputSignals=vehCanInfo.SteerWheelAngle,vehCanInfo.SteerWheelSpd,vehStateCalcInfo.VSCCycFuncSuppAborCond.VSCcycPrefillAborCond,latFuncActiveStateTime.cycPrefillWorkTime
  Bus Selector2 : BusSelector  OutputSignals=latCycTgtCalcInfo.latCycCollisionAssessInfo.latCollisionSeverity,latFuncActiveStateTime.cycPrefillWorkTime
  cycPrefillAborCondFinalJudge : Subsystem
  cycPrefillObjRelAborCondCalc : Subsystem
  cycPrefillSituationRelAborCondCalc : Subsystem
  cycPrefillVehRelAborCondCalc : Subsystem
  cycPrefillAborCondFulfilled : Outport
  wires:
  cycPrefillAborCondFinalJudge/1(cycPrefillAborCondFulfilled) -> cycPrefillAborCondFulfilled/1
  cycPrefillSituationRelAborCondCalc/1(cycPrefillSituationRelAborCondFulfilled) -> cycPrefillAborCondFinalJudge/3(cycPrefillSituationRelAborCondFulfilled)  "AEB_cycPrefillSitRelAborCond"
  cycPrefillVehRelAborCondCalc/1(cycPrefillVehRelAborCondFulfilled) -> cycPrefillAborCondFinalJudge/2(cycPrefillVehRelAborCondFulfilled)  "AEB_cycPrefillVehRelAborCond"
  cycPrefillObjRelAborCondCalc/1(cycPrefillObjRelAborCondFulfilled) -> cycPrefillAborCondFinalJudge/1(cycPrefillObjRelAborCondFulfilled)  "AEB_cycPrefillObjRelAborCond"
  inputSignals/1 -> Bus Selector2/1, Bus Selector1/1, Bus Selector/1
  Bus Selector/1 -> cycPrefillObjRelAborCondCalc/1(ObjectID)  "<ObjectID>"
  Bus Selector1/4 -> cycPrefillVehRelAborCondCalc/4(cycPrefillWorkTime)  "<cycPrefillWorkTime>"
  Bus Selector1/3 -> cycPrefillVehRelAborCondCalc/3(vehStateCalcCycPrefillAborFlg)  "<VSCcycPrefillAborCond>"
  Bus Selector1/2 -> cycPrefillVehRelAborCondCalc/2(SteerWheelSpd)  "<SteerWheelSpd>"
  Bus Selector1/1 -> cycPrefillVehRelAborCondCalc/1(SteerWheelAngle)  "<SteerWheelAngle>"
  Bus Selector2/1 -> cycPrefillSituationRelAborCondCalc/1(CycCollisionSeverity)  "<latCollisionSeverity>"
  Bus Selector2/2 -> cycPrefillSituationRelAborCondCalc/2(cycPrefillWorkTime)  "<cycPrefillWorkTime>"

== system TosAeb/FcwAebJa_Step_sys/ALG_AEBSystem/ALG_AEBController/AEB_FuncCondCalcApp/CycFunctionCondCalc/cycPrefillSuppAborCond/cycPrefillSuppCondCalc ==
  inputSignals : Inport
  Bus Selector : BusSelector  OutputSignals=latCycTgtCalcInfo.latCycObjCalcInfo.latCycObjRawInfo.ObjectID,latCycTgtCalcInfo.latCycObjCalcInfo.latCycObjAbsInfo.vxAbsObj,latCycTgtCalcInfo.latCycObjCalcInfo.latCycObjAbsInfo.ObjFusQuality,latCycTgtCalcInfo.latCycObjCalcInfo.latCycObjRawInfo.dy,latCycTgtCalcInfo.latCycObjCalcInfo.latCycObjRawInfo.vy
  Bus Selector1 : BusSelector  OutputSignals=subjInfo.subjVel,vehConfigInfo.VehConfigIbooster,vehCanInfo.ESP_MasterCylindBrakePressValid,vehCanInfo.ESP_MasterCylindBrakePress,vehCanInfo.IB_BrkPedalAppld,vehCanInfo.SteerWheelAngle,vehCanInfo.SteerWheelSpd,vehCanInfo.AccrPedlPosnDiagc,vehStateCalcInfo.VSCCycFuncSuppAborCond.VSCcycPrefillSuppCond
  Bus Selector2 : BusSelector  OutputSignals=latCycTgtCalcInfo.latCycCollisionAssessInfo.latCollisionSeverity,subjInfo.subjCurvTraj,subjInfo.subjLatAccel,latCycTgtCalcInfo.latCycObjCalcInfo.CollisionRiskProb,latCycTgtCalcInfo.latCycObjCalcInfo.latCycObjRawInfo.ObjectTypeCameraConfirmed,latCycTgtCalcInfo.latCycObjCalcInfo.latCycObjRawInfo.vy,latCycTgtCalcInfo.latCycObjCalcInfo.latCycObjAbsInfo.dxFrontBumper,latCycTgtCalcInfo.latCycCollisionAssessInfo.distBraking4PartBrake,latCycTgtCalcInfo.latCycCollisionAssessInfo.distSecure,subjInfo.subjVel
  cycPrefillObjRelSuppCondCalc : Subsystem
  cycPrefillSituationRelSuppCondCalc : Subsystem
  cycPrefillSuppCondFinalJudge : Subsystem
  cycPrefillVehRelSuppCondCalc : Subsystem
  cycPrefillSuppCondFulfilled : Outport
  wires:
  Bus Selector1/2 -> cycPrefillVehRelSuppCondCalc/2(VehConfigIbooster)  "<VehConfigIbooster>"
  Bus Selector/5 -> cycPrefillObjRelSuppCondCalc/5(vy)  "<vy>"
  Bus Selector/4 -> cycPrefillObjRelSuppCondCalc/4(dy)  "<dy>"
  Bus Selector/3 -> cycPrefillObjRelSuppCondCalc/3(ObjFusQuality)  "<ObjFusQuality>"
  Bus Selector/2 -> cycPrefillObjRelSuppCondCalc/2(vxAbsObj)  "<vxAbsObj>"
  Bus Selector/1 -> cycPrefillObjRelSuppCondCalc/1(ObjectID)  "<ObjectID>"
  Bus Selector1/5 -> cycPrefillVehRelSuppCondCalc/5(IB_BrkPedalAppld)  "<IB_BrkPedalAppld>"
  Bus Selector2/10 -> cycPrefillSituationRelSuppCondCalc/10(subjVel)  "<subjVel>"
  Bus Selector2/9 -> cycPrefillSituationRelSuppCondCalc/9(distSecure)  "<distSecure>"
  Bus Selector2/8 -> cycPrefillSituationRelSuppCondCalc/8(disBraking4PartBrake)  "<distBraking4PartBrake>"
  Bus Selector2/7 -> cycPrefillSituationRelSuppCondCalc/7(dxFrontBumper)  "<dxFrontBumper>"
  Bus Selector2/6 -> cycPrefillSituationRelSuppCondCalc/6(vy)  "<vy>"
  Bus Selector2/5 -> cycPrefillSituationRelSuppCondCalc/5(ObjectTypeCameraConfirmed)  "<ObjectTypeCameraConfirmed>"
  Bus Selector2/4 -> cycPrefillSituationRelSuppCondCalc/4(colliisonRiskProb)  "<CollisionRiskProb>"
  Bus Selector2/3 -> cycPrefillSituationRelSuppCondCalc/3(subjLatAccel)  "<subjLatAccel>"
  Bus Selector2/2 -> cycPrefillSituationRelSuppCondCalc/2(subjCurvTraj)  "<subjCurvTraj>"
  Bus Selector2/1 -> cycPrefillSituationRelSuppCondCalc/1(latCollisionSeverity)  "<latCollisionSeverity>"
  cycPrefillSituationRelSuppCondCalc/1(cycPrefillSituationRelSuppCondFulfilled) -> cycPrefillSuppCondFinalJudge/3(cycPrefillSituationRelSuppCondFulfilled)  "AEB_cycPrefillSitRelSuppCond"
  cycPrefillObjRelSuppCondCalc/1(cycPrefillObjRelSuppCondFulfilled) -> cycPrefillSuppCondFinalJudge/1(cycPrefillObjRelSuppCondFulfilled)  "AEB_cycPrefillObjRelSuppCond"
  cycPrefillVehRelSuppCondCalc/1(cycPrefillVehRelSuppCondFulfilled) -> cycPrefillSuppCondFinalJudge/2(cycPrefillVehRelSuppCondFulfilled)  "AEB_cycPrefillVehRelSuppCond"
  cycPrefillSuppCondFinalJudge/1(cycPrefillSuppCondFulfilled) -> cycPrefillSuppCondFulfilled/1
  inputSignals/1 -> Bus Selector/1, Bus Selector1/1, Bus Selector2/1
  Bus Selector1/7 -> cycPrefillVehRelSuppCondCalc/7(SteerWheelSpd)  "<SteerWheelSpd>"
  Bus Selector1/8 -> cycPrefillVehRelSuppCondCalc/8(AccrCyclPosnDiagc)  "<AccrPedlPosnDiagc>"
  Bus Selector1/6 -> cycPrefillVehRelSuppCondCalc/6(SteerWheelAngle)  "<SteerWheelAngle>"
  Bus Selector1/4 -> cycPrefillVehRelSuppCondCalc/4(ESP_MasterCylindBrakePress)  "<ESP_MasterCylindBrakePress>"
  Bus Selector1/3 -> cycPrefillVehRelSuppCondCalc/3(ESP_MasterCylindBrakePressValid)  "<ESP_MasterCylindBrakePressValid>"
  Bus Selector1/9 -> cycPrefillVehRelSuppCondCalc/9(vehStateCalcCycPrefillSuppFlg)  "<VSCcycPrefillSuppCond>"
  Bus Selector1/1 -> cycPrefillVehRelSuppCondCalc/1(subjVel)  "<subjVel>"

== system TosAeb/FcwAebJa_Step_sys/ALG_AEBSystem/ALG_AEBController/AEB_FuncCondCalcApp/JaCycFunctionCondCalc/JaCycAEBfullSuppAborCond/JaCycAEBfullAborCondCalc ==
  inputSignals : Inport
  Bus Selector : BusSelector  OutputSignals=JaCycTgtCalcInfo.JACycTgtObjInfo.JaCycObj.ObjectID
  Bus Selector1 : BusSelector  OutputSignals=vehCanInfo.SteerWheelSpd,vehCanInfo.AccrPedlPosnDiagc,vehStateCalcInfo.VSC_JAFuncSuppAborCond.VSC_JACycAEBfullAborCond
  JaCycAEBfullAborCondFinalJudge : Subsystem
  JaCycAEBfullObjRelAborCondCalc : Subsystem
  JaCycAEBfullVehRelAborCondCalc : Subsystem
  JaCycAEBfullAborCondNoStandstillFulfilled : Outport
  JaCycAEBfullAborCondStandstillFulfilled : Outport  Port=2
  wires:
  JaCycAEBfullAborCondFinalJudge/2(JaCycAEBfullAborCondStndstillFulfilled) -> JaCycAEBfullAborCondStandstillFulfilled/1
  Bus Selector1/3 -> JaCycAEBfullVehRelAborCondCalc/3(VSC_JaCycAEBfullAborCond)  "<VSC_JACycAEBfullAborCond>"
  Bus Selector/1 -> JaCycAEBfullObjRelAborCondCalc/1(ObjectID)  "<ObjectID>"
  inputSignals/1 -> Bus Selector/1, Bus Selector1/1
  JaCycAEBfullObjRelAborCondCalc/1(JaCycAEBfullObjRelAborCond) -> JaCycAEBfullAborCondFinalJudge/1(JaCycAEBfullObjRelAborCond)  "AEB_JACycAEBfullObjRelAborCond"
  JaCycAEBfullVehRelAborCondCalc/1(JaCycAEBfullVehRelAborCond) -> JaCycAEBfullAborCondFinalJudge/2(JaCycAEBfullVehRelAborCond)  "AEB_JaCycAEBfullVehRelAborCond"
  JaCycAEBfullAborCondFinalJudge/1(JaCycAEBfullAborCondNoStndstillFulfilled) -> JaCycAEBfullAborCondNoStandstillFulfilled/1
  Bus Selector1/1 -> JaCycAEBfullVehRelAborCondCalc/1(SteerWheelSpd_DegS)  "<SteerWheelSpd>"
  Bus Selector1/2 -> JaCycAEBfullVehRelAborCondCalc/2(AccelPedalPositionDiag)  "<AccrPedlPosnDiagc>"

== system TosAeb/FcwAebJa_Step_sys/ALG_AEBSystem/ALG_AEBController/AEB_FuncCondCalcApp/JaCycFunctionCondCalc/JaCycAEBfullSuppAborCond/JaCycAEBfullSuppCondCalc ==
  inputSignals : Inport
  Bus Selector : BusSelector  OutputSignals=JaCycTgtCalcInfo.JACycTgtObjInfo.JaCycObj.ObjectMovingOrientation,JaCycTgtCalcInfo.JACycTgtObjInfo.JaCycObj.ObjectID,JaCycTgtCalcInfo.JACycTgtObjInfo.JaCycObj.ObjectExistProb,JaCycTgtCalcInfo.JACycTgtObjInfo.JaCycObj.ObjectIsObstacleProb,JaCycTgtCalcInfo.JACycTgtObjInfo.JaCycObj.ObjectTypeCameraConfirmed,JaCycTgtCalcInfo.JACycTgtObjInfo.JaCycObj.dy,JaCycTgtCalcInfo.JACycTgtObjInfo.JaCycObjAbsInfo.vxAbsObj,JaCycTgtCalcInfo.JACycTgtObjInfo.JaCycObjAbsInfo.vyRelCorr,JaCycTgtCalcInfo.JACycTgtObjInfo.JaCycObjAbsInfo.ObjFusQuality
  Bus Selector1 : BusSelector  OutputSignals=subjInfo.subjVel,vehConfigInfo.VehConfigIbooster,vehCanInfo.ESP_MasterCylindBrakePress,vehCanInfo.AccrPedlPosnDiagc,vehCanInfo.SteerWheelAngle_Deg,vehCanInfo.LeftTurnSWSts,vehCanInfo.RightTurnSWSts,subjInfo.subjLatAccel,vehStateCalcInfo.VSC_JAFuncSuppAborCond.VSC_JACycAEBfullSuppCond
  Bus Selector2 : BusSelector  OutputSignals=JaCycTgtCalcInfo.JACycCollisonAssessInfo.distBraking,JaCycTgtCalcInfo.JACycCollisonAssessInfo.distRadical2Collsion,JaCycTgtCalcInfo.JACycTgtObjInfo.JaCycObjColliInfo.tPredictTime,JaCycTgtCalcInfo.JACycCollisonAssessInfo.TTB,JaCycTgtCalcInfo.JACycTgtObjInfo.JaCycObjColliInfo.ObjColliFlg
  JaCycAEBfullSuppCondFinalJudge : Subsystem
  JaCycAEBfullSuppObjCondCalc : Subsystem
  JaCycAEBfullSuppSitCondCalc : Subsystem
  JaCycAEBfullSuppVehCondCalc : Subsystem
  JaCycAEBfullSuppCond : Outport
  wires:
  Bus Selector1/9 -> JaCycAEBfullSuppVehCondCalc/9(VSC_JaCycAEBfullSuppCond)  "<VSC_JACycAEBfullSuppCond>"
  inputSignals/1 -> Bus Selector2/1, Bus Selector1/1, Bus Selector/1
  JaCycAEBfullSuppCondFinalJudge/1(JaCycAEBfullSuppCond) -> JaCycAEBfullSuppCond/1
  JaCycAEBfullSuppVehCondCalc/1(JaCycAEBfullVehActiveCond) -> JaCycAEBfullSuppCondFinalJudge/2(JaCycAEBfullVehRelActiveCond)  "AEB_JaCycAEBfullVehActiveCond"
  JaCycAEBfullSuppObjCondCalc/1(JaCycAEBfullObjActiveCond) -> JaCycAEBfullSuppCondFinalJudge/1(JaCycAEBfullObjRelActiveCond)  "AEB_JaCycAEBfullObjActiveCond"
  JaCycAEBfullSuppSitCondCalc/1(JaCycAEBfullSuppSitActiveCond) -> JaCycAEBfullSuppCondFinalJudge/3(JaCycAEBfullSitRelActiveCond)  "AEB_JACycAEBfullSitActiveCond"
  Bus Selector/1 -> JaCycAEBfullSuppObjCondCalc/1(ObjectMovingOrientation)  "<ObjectMovingOrientation>"
  Bus Selector/2 -> JaCycAEBfullSuppObjCondCalc/2(ObjectID)  "<ObjectID>"
  Bus Selector/3 -> JaCycAEBfullSuppObjCondCalc/3(ObjectExistProb)  "<ObjectExistProb>"
  Bus Selector/4 -> JaCycAEBfullSuppObjCondCalc/4(ObjectIsObstacleProb)  "<ObjectIsObstacleProb>"
  Bus Selector/5 -> JaCycAEBfullSuppObjCondCalc/5(ObjectTypeCameraConfirmed)  "<ObjectTypeCameraConfirmed>"
  Bus Selector/7 -> JaCycAEBfullSuppObjCondCalc/7(vxAbsObj)  "<vxAbsObj>"
  Bus Selector/8 -> JaCycAEBfullSuppObjCondCalc/8(vyRelCorr)  "<vyRelCorr>"
  Bus Selector1/1 -> JaCycAEBfullSuppVehCondCalc/1(subjVel)  "<subjVel>"
  Bus Selector1/3 -> JaCycAEBfullSuppVehCondCalc/3(ESP_MasterCylindBrakePress)  "<ESP_MasterCylindBrakePress>"
  Bus Selector1/4 -> JaCycAEBfullSuppVehCondCalc/4(AccrPedlPosnDiagc)  "<AccrPedlPosnDiagc>"
  Bus Selector1/5 -> JaCycAEBfullSuppVehCondCalc/5(SteerWheelAngle_Deg)  "<SteerWheelAngle_Deg>"
  Bus Selector1/6 -> JaCycAEBfullSuppVehCondCalc/6(LeftTurnSWSts)  "<LeftTurnSWSts>"
  Bus Selector1/8 -> JaCycAEBfullSuppVehCondCalc/8(subjLatAccel)  "<subjLatAccel>"
  Bus Selector1/7 -> JaCycAEBfullSuppVehCondCalc/7(RightTurnSWSts)  "<RightTurnSWSts>"
  Bus Selector2/3 -> JaCycAEBfullSuppSitCondCalc/3(tPredictTime)  "<tPredictTime>"
  Bus Selector2/2 -> JaCycAEBfullSuppSitCondCalc/2(distRadical2Collsion)  "<distRadical2Collsion>"
  Bus Selector2/4 -> JaCycAEBfullSuppSitCondCalc/4(TTB)  "<TTB>"
  Bus Selector2/1 -> JaCycAEBfullSuppSitCondCalc/1(distBraking)  "<distBraking>"
  Bus Selector2/5 -> JaCycAEBfullSuppSitCondCalc/5(ObjColliFlg)  "<ObjColliFlg>"
  Bus Selector/6 -> JaCycAEBfullSuppObjCondCalc/6(dy)  "<dy>"
  Bus Selector/9 -> JaCycAEBfullSuppObjCondCalc/9(ObjFusQuality)  "<ObjFusQuality>"
  Bus Selector1/2 -> JaCycAEBfullSuppVehCondCalc/2(VehConfigIbooster)  "<VehConfigIbooster>"

== system TosAeb/FcwAebJa_Step_sys/ALG_AEBSystem/ALG_AEBController/AEB_FuncCondCalcApp/JaCycFunctionCondCalc/JaCycPrefillSuppAborCond/JaCycPrefillAborCondCalc ==
  inputSignals : Inport
  Bus Selector : BusSelector  OutputSignals=JaCycTgtCalcInfo.JACycTgtObjInfo.JaCycObj.ObjectID
  Bus Selector1 : BusSelector  OutputSignals=vehCanInfo.SteerWheelSpd_DegS,vehCanInfo.AccrPedlPosnDiagc,vehStateCalcInfo.VSC_JAFuncSuppAborCond.VSC_JACycPrefillAborCond
  JaCycPrefillAborCondFinalJudge : Subsystem
  JaCycPrefillObjRelAborCondCalc : Subsystem
  JaCycPrefillSitRelAborCondCalc : Subsystem
  JaCycPrefillVehRelAborCondCalc : Subsystem
  JaCycPrefillAborCond : Outport
  wires:
  Bus Selector1/2 -> JaCycPrefillVehRelAborCondCalc/2(AccelPedalPositionDiag)  "<AccrPedlPosnDiagc>"
  Bus Selector1/1 -> JaCycPrefillVehRelAborCondCalc/1(SteerWheelSpd_DegS)  "<SteerWheelSpd_DegS>"
  JaCycPrefillAborCondFinalJudge/1(JaCycPrefillAborCond) -> JaCycPrefillAborCond/1
  JaCycPrefillSitRelAborCondCalc/1(JaCycPrefillSitRelAborCond) -> JaCycPrefillAborCondFinalJudge/3(JaCycPrefillSitRelAborCond)  "AEB_JaCycPrefillSitRelAborCond"
  JaCycPrefillVehRelAborCondCalc/1(JaCycPrefillVehRelAborCond) -> JaCycPrefillAborCondFinalJudge/2(JaCycPrefillVehRelAborCond)  "AEB_JaCycPrefillVehRelAborCond"
  JaCycPrefillObjRelAborCondCalc/1(JaCycPrefillObjRelAborCond) -> JaCycPrefillAborCondFinalJudge/1(JaCycPrefillObjRelAborCond)  "AEB_JaCycPrefillObjRelAborCond"
  inputSignals/1 -> Bus Selector1/1, Bus Selector/1
  Bus Selector/1 -> JaCycPrefillObjRelAborCondCalc/1(ObjectID)  "<ObjectID>"
  Bus Selector1/3 -> JaCycPrefillVehRelAborCondCalc/3(VSC_JaCycPrefillAborCond)  "<VSC_JACycPrefillAborCond>"

== system TosAeb/FcwAebJa_Step_sys/ALG_AEBSystem/ALG_AEBController/AEB_FuncCondCalcApp/JaCycFunctionCondCalc/JaCycPrefillSuppAborCond/JaCycPrefillSuppCondCalc ==
  inputSignals : Inport
  Bus Selector : BusSelector  OutputSignals=JaCycTgtCalcInfo.JACycTgtObjInfo.JaCycObj.ObjectMovingOrientation,JaCycTgtCalcInfo.JACycTgtObjInfo.JaCycObj.ObjectID,JaCycTgtCalcInfo.JACycTgtObjInfo.JaCycObj.ObjectExistProb,JaCycTgtCalcInfo.JACycTgtObjInfo.JaCycObj.ObjectIsObstacleProb,JaCycTgtCalcInfo.JACycTgtObjInfo.JaCycObj.ObjectTypeCameraConfirmed,JaCycTgtCalcInfo.JACycTgtObjInfo.JaCycObj.dy,JaCycTgtCalcInfo.JACycTgtObjInfo.JaCycObjAbsInfo.vxAbsObj,JaCycTgtCalcInfo.JACycTgtObjInfo.JaCycObjAbsInfo.vyRelCorr,JaCycTgtCalcInfo.JACycTgtObjInfo.JaCycObjAbsInfo.ObjFusQuality
  Bus Selector1 : BusSelector  OutputSignals=subjInfo.subjVel,vehConfigInfo.VehConfigIbooster,vehCanInfo.BrakePedalStatus_G,vehCanInfo.ESP_MasterCylindBrakePress,vehCanInfo.IB_BrkPedalAppld,vehCanInfo.AccrPedlPosnDiagc,vehCanInfo.SteerWheelAngle_Deg,vehCanInfo.LeftTurnSWSts,vehCanInfo.RightTurnSWSts,subjInfo.subjLatAccel,vehStateCalcInfo.VSC_JAFuncSuppAborCond.VSC_JACycPrefillSuppCond
  Bus Selector2 : BusSelector  OutputSignals=JaCycTgtCalcInfo.JACycCollisonAssessInfo.distBraking,JaCycTgtCalcInfo.JACycCollisonAssessInfo.distRadical2Collsion,JaCycTgtCalcInfo.JACycTgtObjInfo.JaCycObjColliInfo.tPredictTime,JaCycTgtCalcInfo.JACycCollisonAssessInfo.TTB,JaCycTgtCalcInfo.JACycTgtObjInfo.JaCycObjColliInfo.ObjColliFlg
  JaCycPrefillSuppCondFinalJudge : Subsystem
  JaCycPrefillSuppObjCondCalc : Subsystem
  JaCycPrefillSuppSitCondCalc : Subsystem
  JaCycPrefillSuppVehCondCalc : Subsystem
  JaCycPrefillSuppCond : Outport
  wires:
  Bus Selector1/11 -> JaCycPrefillSuppVehCondCalc/11(VSC_JaCycPrefillSuppCond)  "<VSC_JACycPrefillSuppCond>"
  Bus Selector2/5 -> JaCycPrefillSuppSitCondCalc/5(ObjColliFlg)  "<ObjColliFlg>"
  Bus Selector2/4 -> JaCycPrefillSuppSitCondCalc/4(TTB)  "<TTB>"
  Bus Selector2/3 -> JaCycPrefillSuppSitCondCalc/3(tPredictTime)  "<tPredictTime>"
  Bus Selector1/10 -> JaCycPrefillSuppVehCondCalc/10(subjLatAccel)  "<subjLatAccel>"
  Bus Selector1/8 -> JaCycPrefillSuppVehCondCalc/8(LeftTurnSWSts)  "<LeftTurnSWSts>"
  Bus Selector1/7 -> JaCycPrefillSuppVehCondCalc/7(SteerWheelAngle_Deg)  "<SteerWheelAngle_Deg>"
  Bus Selector1/6 -> JaCycPrefillSuppVehCondCalc/6(AccrPedlPosnDiagc)  "<AccrPedlPosnDiagc>"
  Bus Selector1/4 -> JaCycPrefillSuppVehCondCalc/4(ESP_MasterCylindBrakePress)  "<ESP_MasterCylindBrakePress>"
  Bus Selector1/3 -> JaCycPrefillSuppVehCondCalc/3(BrakePedalStatus_G)  "<BrakePedalStatus_G>"
  Bus Selector1/1 -> JaCycPrefillSuppVehCondCalc/1(subjVel)  "<subjVel>"
  Bus Selector/8 -> JaCycPrefillSuppObjCondCalc/8(vyRelCorr)  "<vyRelCorr>"
  Bus Selector/7 -> JaCycPrefillSuppObjCondCalc/7(vxAbsObj)  "<vxAbsObj>"
  Bus Selector/5 -> JaCycPrefillSuppObjCondCalc/5(ObjectTypeCameraConfirmed)  "<ObjectTypeCameraConfirmed>"
  Bus Selector/4 -> JaCycPrefillSuppObjCondCalc/4(ObjectIsObstacleProb)  "<ObjectIsObstacleProb>"
  Bus Selector/3 -> JaCycPrefillSuppObjCondCalc/3(ObjectExistProb)  "<ObjectExistProb>"
  Bus Selector/2 -> JaCycPrefillSuppObjCondCalc/2(ObjectID)  "<ObjectID>"
  Bus Selector/1 -> JaCycPrefillSuppObjCondCalc/1(ObjectMovingOrientation)  "<ObjectMovingOrientation>"
  JaCycPrefillSuppSitCondCalc/1(AEBJAVehPrefillSuppSitActiveCond) -> JaCycPrefillSuppCondFinalJudge/3(JaCycPrefillSitActvCond)  "AEB_JaCycPrefillSitActiveCond"
  JaCycPrefillSuppObjCondCalc/1(JaCycPrefillSuppObjActiveCond) -> JaCycPrefillSuppCondFinalJudge/1(JaCycPrefillObjActvCond)  "AEB_JaCycPrefillObjActiveCond"
  JaCycPrefillSuppVehCondCalc/1(JaCycPrefillVehActiveCond) -> JaCycPrefillSuppCondFinalJudge/2(JaCycPrefillVehActvCond)  "AEB_JaCycPrefillVehActiveCond"
  JaCycPrefillSuppCondFinalJudge/1(JaCycPrefillSuppCond) -> JaCycPrefillSuppCond/1
  inputSignals/1 -> Bus Selector2/1, Bus Selector1/1, Bus Selector/1
  Bus Selector1/9 -> JaCycPrefillSuppVehCondCalc/9(RightTurnSWSts)  "<RightTurnSWSts>"
  Bus Selector2/2 -> JaCycPrefillSuppSitCondCalc/2(distRadical2Collsion)  "<distRadical2Collsion>"
  Bus Selector2/1 -> JaCycPrefillSuppSitCondCalc/1(distBraking)  "<distBraking>"
  Bus Selector1/5 -> JaCycPrefillSuppVehCondCalc/5(IB_BrkPedalAppld)  "<IB_BrkPedalAppld>"
  Bus Selector/6 -> JaCycPrefillSuppObjCondCalc/6(dy)  "<dy>"
  Bus Selector/9 -> JaCycPrefillSuppObjCondCalc/9(ObjFusQuality)  "<ObjFusQuality>"
  Bus Selector1/2 -> JaCycPrefillSuppVehCondCalc/2(VehConfigIbooster)  "<VehConfigIbooster>"
